simulink model "wlan_phy_rx_pmd"  (Simulink 7.8)
settings: Solver=VariableStepDiscrete  SolverMode=SingleTasking  StartTime=0.0  StopTime=rx_sim.sim_time  RelTol=1e-3
PostLoadFcn:
  | wlan_phy_rx_init
InitFcn:
  | wlan_phy_rx_init
StartFcn:
  | wlan_phy_rx_init
values from neighbouring files (symbols this model uses):
  barker_seq20 = 0.95 * circshift(barker_seq20, [0 4]) ./ max(abs(barker_seq20))    [wlan_phy_rx_init.m]
  bit_scrambler_lfsr_bytes = sum(bit_scrambler_lfsr_bytes .* repmat(2.^[0:7], 127, 1),2)    [wlan_phy_rx_init.m]
  longCorr_coef_i = [3*real(longCorr_coef)]    [wlan_phy_rx_init.m]
  longCorr_coef_q = [3*imag(longCorr_coef)]    [wlan_phy_rx_init.m]
  MAX_CP_LEN = 32    [wlan_phy_rx_init.m]
  MAX_NCBPS = NCBPS_11n_64QAM    (= 312)    [wlan_phy_rx_init.m]
  MAX_NUM_BYTES = 2^16-1    (= 65535)    [wlan_phy_rx_init.m]
  MAX_NUM_SC = 64    [wlan_phy_rx_init.m]
  MAX_NUM_SYMS = 600    [wlan_phy_rx_init.m]
  NDBPS_11a_BPSK12 = 24    [wlan_phy_rx_init.m]
  preFFT_sampBuff_numSamps = 4*MAX_NUM_SC    (= 256)    [wlan_phy_rx_init.m]
  rx_sim = struct()    [wlan_phy_rx_init.m]
  scr_ind_rev = zeros(1,128)    [wlan_phy_rx_init.m]
undefined symbols (not defined in the file or in its folder): gcbh, mcs_rom_11ag, mcs_rom_11n, wlan_rx_deinterleave_rom_gen, xlBlockLoadCallback, xlGetNormalizedPeriod, xlipgetpartsetting
libraries: xbsIndex_r4 x4943, xbsEDKLib_r4 x1
(part 11 of 28)

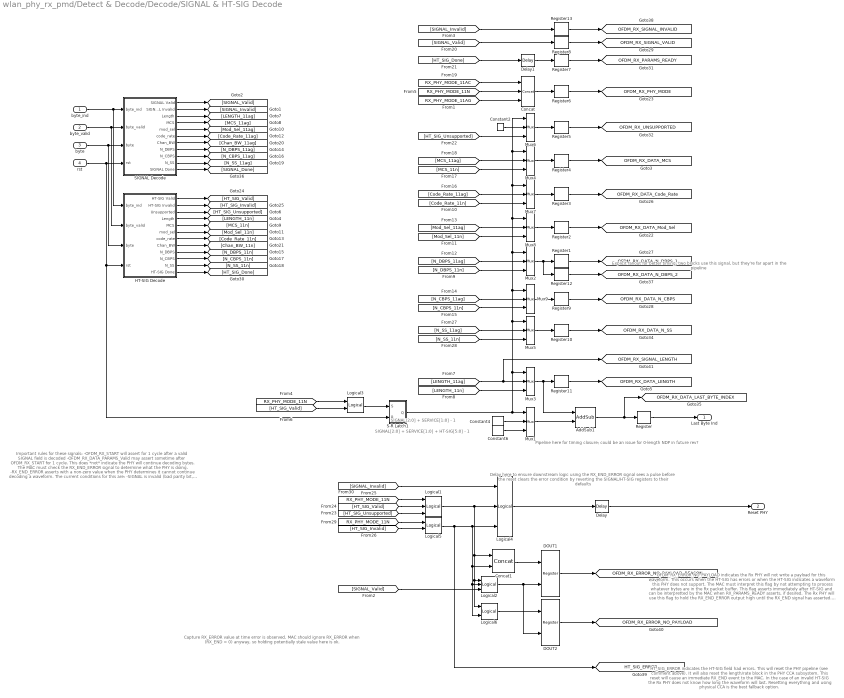
== system wlan_phy_rx_pmd/Detect & Decode/Decode/SIGNAL & HT-SIG Decode ==
  note: SIGNAL[2:0] + SERVICE[1:0] - 1
  note: SIGNAL[2:0] + SERVICE[1:0] +
      | HT-SIG[5:0] - 1
  note: OFDM_RX_ERROR_NO_PAYLOAD indicates the Rx PHY
      | will not write a payload for this waveform. This occurs when
      | the HT-SIG has errors or when the HT-SIG indicates a
      | waveform this PHY does not support. The MAC must interpret
      | this flag by not attempting to process whatever bytes are in
      | the Rx packet buffer.
      |
      | This flag asserts immediately after HT-SIG and can be interpretted
      | by the MAC when RX_PARAMS_READY asserts, if desired.
      |
      | The Rx PHY will use this flag to hold the RX_END_ERROR output
      | high until the RX_END signal has asserted. The MAC uses RX_END
      | to qualify RX_END_ERROR.
  note: Important rules for these signals:
      | -OFDM_RX_START will assert for 1 cycle after a valid SIGNAL field is decoded
      | -OFDM_RX_DATA_PARAMS_Valid may assert sometime after OFDM_RX_START for 1 cycle. This
      |   does *not* indicate the PHY will continue decoding bytes. The MAC must check the RX_END_ERROR
      |   signal to determine what the PHY is doing.
      | -RX_END_ERROR asserts with a non-zero value when the PHY determines it cannot continue decoding
      |   a waveform. The current conditions for this are:
      |    -SIGNAL is invalid (bad parity bit, invalid RATE or LENGTH value)
      |    -HT-SIG is invalid (bad MCS, bad TAIL or bad CRC)
      |    -HT-SIG.MCS is unsupported
  note: Delay here to ensure downstream logic using
      | the RX_END_ERROR signal sees a pulse before
      | the reset clears the error condition by reverting
      | the SIGNAL/HT-SIG registers to their defaults
  note: Capture RX_ERROR value at time error is observed. MAC should
      | ignore RX_ERROR when (RX_END = 0) anyway, so
      | holding potentially stale value here is ok.
  note: Pipeline here for timing closure; could be
      | an issue for 0-length NDP in future rev?
  note: Explicit fanout for better timing; two blocks
      | use this signal, but they're far apart in the pipeline
  note: HT_SIG_ERROR indicates the HT-SIG field had errors. This will reset
      | the PHY pipeline (see comment above). It will also reset the length/rate
      | block in the PHY CCA subsystem. This reset will cause an immediate RX_END
      | event to the MAC. In the case of an invalid HT-SIG the Rx PHY does not know
      | how long the waveform will last. Resetting everything and using physical CCA
      | is the best fallback option.
  byte_ind : Inport
  byte_valid : Inport  Port=2
  byte : Inport  Port=3
  rst : Inport  Port=4
  AddSub1 : xbsIndex_r4/AddSub  mode=Addition  use_carryin=off  use_carryout=off  en=off  latency=0  precision=Full  arith_type="Signed  (2's comp)"  n_bits=ceil(log2(MAX_NUM_BYTES))  bin_pt=0  quantization=Truncate  overflow=Wrap  dbl_ovrd=off  use_behavioral_HDL=off  hw_selection=Fabric  pipelined=off  block_type=addsub
  Concat : xbsIndex_r4/Concat  num_inputs=3  dbl_ovrd=off  block_type=concat
  Concat1 : xbsIndex_r4/Concat  num_inputs=2  dbl_ovrd=off  block_type=concat
  Constant2 : xbsIndex_r4/Constant  const=0  gui_display_data_type=Boolean  arith_type=Boolean  n_bits=2  bin_pt=0  preci_type=Single  exp_width=8  frac_width=24  explicit_period=off  period=1  equ=P=C  opselect=C  inp2=PCIN>>17  opr=+  inp1=P  carry=CIN  dbl_ovrd=off  block_type=constant
  Constant4 : xbsIndex_r4/Constant  const=4  gui_display_data_type=Fixed-point  arith_type=Unsigned  n_bits=3  bin_pt=0  preci_type=Single  exp_width=8  frac_width=24  explicit_period=off  period=1  equ=P=C  opselect=C  inp2=PCIN>>17  opr=+  inp1=P  carry=CIN  dbl_ovrd=off  block_type=constant
  Constant6 : xbsIndex_r4/Constant  const=4+6  gui_display_data_type=Fixed-point  arith_type=Unsigned  n_bits=4  bin_pt=0  preci_type=Single  exp_width=8  frac_width=24  explicit_period=off  period=1  equ=P=C  opselect=C  inp2=PCIN>>17  opr=+  inp1=P  carry=CIN  dbl_ovrd=off  block_type=constant
  DOUT1 : xbsIndex_r4/Register  init=0  rst=off  en=on  dbl_ovrd=off  block_type=register
  DOUT2 : xbsIndex_r4/Register  init=0  rst=off  en=on  dbl_ovrd=off  block_type=register
  Delay : xbsIndex_r4/Delay  rst=off  infoeditControl=Selection of Reset will increase slice count due to use of real FFs and instead of SRLs  en=off  latency=4  dbl_ovrd=off  reg_retiming=off  block_type=delay
  Delay1 : xbsIndex_r4/Delay  rst=off  infoeditControl=Selection of Reset will increase slice count due to use of real FFs and instead of SRLs  en=off  latency=1  dbl_ovrd=off  reg_retiming=off  block_type=delay
  HT-SIG Decode : Subsystem
  Logical1 : xbsIndex_r4/Logical  logical_function=AND  inputs=3  en=off  latency=0  precision=Full  arith_type=Unsigned  n_bits=16  bin_pt=0  align_bp=on  dbl_ovrd=off  block_type=logical
  Logical2 : xbsIndex_r4/Logical  logical_function=OR  inputs=3  en=off  latency=0  precision=Full  arith_type=Unsigned  n_bits=16  bin_pt=0  align_bp=on  dbl_ovrd=off  block_type=logical
  Logical3 : xbsIndex_r4/Logical  logical_function=AND  inputs=2  en=off  latency=0  precision=Full  arith_type=Unsigned  n_bits=16  bin_pt=0  align_bp=on  dbl_ovrd=off  block_type=logical
  Logical4 : xbsIndex_r4/Logical  logical_function=OR  inputs=3  en=off  latency=0  precision=Full  arith_type=Unsigned  n_bits=16  bin_pt=0  align_bp=on  dbl_ovrd=off  block_type=logical
  Logical5 : xbsIndex_r4/Logical  logical_function=AND  inputs=2  en=off  latency=0  precision=Full  arith_type=Unsigned  n_bits=16  bin_pt=0  align_bp=on  dbl_ovrd=off  block_type=logical
  Logical6 : xbsIndex_r4/Logical  logical_function=OR  inputs=2  en=off  latency=0  precision=Full  arith_type=Unsigned  n_bits=16  bin_pt=0  align_bp=on  dbl_ovrd=off  block_type=logical
  Mux{1..9} (9 alike) : xbsIndex_r4/Mux  inputs=2  en=off  latency=0  precision=Full  arith_type=Unsigned  n_bits=16  bin_pt=14  quantization=Truncate  overflow=Wrap  dbl_ovrd=off  block_type=mux
  Register{,1..13} (14 alike) : xbsIndex_r4/Register  init=0  rst=off  en=off  dbl_ovrd=off  block_type=register
  S-R Latch1 : Subsystem
  SIGNAL Decode : Subsystem
  Last Byte Ind : Outport
  Reset PHY : Outport  Port=2
  tag scope: HT_SIG_ERROR=global, OFDM_RX_DATA_Code_Rate=global, OFDM_RX_DATA_LAST_BYTE_INDEX=global, OFDM_RX_DATA_LENGTH=global, OFDM_RX_DATA_MCS=global, OFDM_RX_DATA_Mod_Sel=global, OFDM_RX_DATA_N_CBPS=global, OFDM_RX_DATA_N_DBPS_1=global, OFDM_RX_DATA_N_DBPS_2=global, OFDM_RX_DATA_N_SS=global, OFDM_RX_ERROR_NO_PAYLOAD=global, OFDM_RX_ERROR_NO_PAYLOAD_REASON=global, OFDM_RX_PARAMS_READY=global, OFDM_RX_PHY_MODE=global, OFDM_RX_SIGNAL_INVALID=global, OFDM_RX_SIGNAL_LENGTH=global, OFDM_RX_SIGNAL_VALID=global, OFDM_RX_UNSUPPORTED=global
  wires:
  byte_ind/1 -> SIGNAL Decode/1(byte_ind), HT-SIG Decode/1(byte_ind)
  byte_valid/1 -> SIGNAL Decode/2(byte_valid), HT-SIG Decode/2(byte_valid)
  byte/1 -> SIGNAL Decode/3(byte), HT-SIG Decode/3(byte)
  rst/1 -> SIGNAL Decode/4(rst), HT-SIG Decode/4(rst), S-R Latch1/2(R)
  SIGNAL Decode/3(Length) -> [LENGTH_11ag]
  SIGNAL Decode/4(MCS) -> [MCS_11ag]
  SIGNAL Decode/5(mod_sel) -> [Mod_Sel_11ag]
  SIGNAL Decode/1(SIGNAL Valid) -> [SIGNAL_Valid]
  SIGNAL Decode/2(SIGNAL Invalid) -> [SIGNAL_Invalid]
  SIGNAL Decode/6(code_rate) -> [Code_Rate_11ag]
  SIGNAL Decode/7(Chan_BW) -> [Chan_BW_11ag]
  SIGNAL Decode/8(N_DBPS) -> [N_DBPS_11ag]
  SIGNAL Decode/9(N_CBPS) -> [N_CBPS_11ag]
  SIGNAL Decode/10(N_SS) -> [N_SS_11ag]
  HT-SIG Decode/1(HT-SIG Valid) -> [HT_SIG_Valid]
  HT-SIG Decode/2(HT-SIG Invalid) -> [HT_SIG_Invalid]
  HT-SIG Decode/3(Unsupported) -> [HT_SIG_Unsupported]
  HT-SIG Decode/4(Length) -> [LENGTH_11n]
  HT-SIG Decode/5(MCS) -> [MCS_11n]
  HT-SIG Decode/6(mod_sel) -> [Mod_Sel_11n]
  HT-SIG Decode/7(code_rate) -> [Code_Rate_11n]
  HT-SIG Decode/8(Chan_BW) -> [Chan_BW_11n]
  HT-SIG Decode/9(N_DBPS) -> [N_DBPS_11n]
  HT-SIG Decode/10(N_CBPS) -> [N_CBPS_11n]
  HT-SIG Decode/11(N_SS) -> [N_SS_11n]
  Mux3/1 -> AddSub1/1, Register11/1
  AddSub1/1 -> [OFDM_RX_DATA_LAST_BYTE_INDEX], Register/1
  [LENGTH_11ag] -> Mux3/2, [OFDM_RX_SIGNAL_LENGTH]
  [LENGTH_11n] -> Mux3/3
  [Code_Rate_11ag] -> Mux7/2
  [Code_Rate_11n] -> Mux7/3
  Mux7/1 -> Register3/1
  [Mod_Sel_11ag] -> Mux8/2
  [Mod_Sel_11n] -> Mux8/3
  Mux8/1 -> Register2/1
  [N_DBPS_11ag] -> Mux2/2
  [N_DBPS_11n] -> Mux2/3
  Mux2/1 -> Register1/1, Register12/1
  [N_CBPS_11ag] -> Mux9/2
  [N_CBPS_11n] -> Mux9/3
  Mux9/1 -> Register9/1
  Mux1/1 -> AddSub1/2
  Constant4/1 -> Mux1/2
  Constant6/1 -> Mux1/3
  Logical3/1 -> S-R Latch1/1(S)
  S-R Latch1/1(Q) -> Mux1/1, Mux3/1, Mux9/1, Mux2/1, Mux8/1, Mux7/1, Mux4/1, Mux6/1, Mux5/1
  [MCS_11ag] -> Mux4/2
  [MCS_11n] -> Mux4/3
  Mux4/1 -> Register4/1
  [RX_PHY_MODE_11AC] -> Concat/1
  [RX_PHY_MODE_11N] -> Concat/2
  [RX_PHY_MODE_11AG] -> Concat/3
  Concat/1 -> Register6/1
  [SIGNAL_Valid] -> Register8/1
  HT-SIG Decode/12(HT-SIG Done) -> [HT_SIG_Done]
  [HT_SIG_Done] -> Delay1/1
  Delay1/1 -> Register7/1
  [RX_PHY_MODE_11N] -> Logical1/1
  [HT_SIG_Valid] -> Logical1/2
  [SIGNAL_Invalid] -> Logical4/1
  Logical5/1 -> Logical4/3, Concat1/2, Logical2/2, Logical6/2, [HT_SIG_ERROR]
  Logical4/1 -> Delay/1
  [N_SS_11ag] -> Mux5/2
  [N_SS_11n] -> Mux5/3
  Mux5/1 -> Register10/1
  [HT_SIG_Unsupported] -> Mux6/3
  Constant2/1 -> Mux6/2
  Mux6/1 -> Register5/1
  [RX_PHY_MODE_11N] -> Logical5/1
  [HT_SIG_Invalid] -> Logical5/2
  Logical1/1 -> Logical4/2, Concat1/1, Logical2/1, Logical6/1
  [HT_SIG_Unsupported] -> Logical1/3
  SIGNAL Decode/11(SIGNAL Done) -> [SIGNAL_Done]
  Concat1/1 -> DOUT1/1
  Delay/1 -> Reset PHY/1
  Logical2/1 -> DOUT1/2, DOUT2/2
  DOUT1/1 -> [OFDM_RX_ERROR_NO_PAYLOAD_REASON]
  [SIGNAL_Valid] -> Logical2/3
  Register/1 -> Last Byte Ind/1
  Register1/1 -> [OFDM_RX_DATA_N_DBPS_1]
  Register2/1 -> [OFDM_RX_DATA_Mod_Sel]
  Register3/1 -> [OFDM_RX_DATA_Code_Rate]
  Register4/1 -> [OFDM_RX_DATA_MCS]
  Register5/1 -> [OFDM_RX_UNSUPPORTED]
  Register6/1 -> [OFDM_RX_PHY_MODE]
  Register7/1 -> [OFDM_RX_PARAMS_READY]
  Register8/1 -> [OFDM_RX_SIGNAL_VALID]
  Register9/1 -> [OFDM_RX_DATA_N_CBPS]
  Register10/1 -> [OFDM_RX_DATA_N_SS]
  Register11/1 -> [OFDM_RX_DATA_LENGTH]
  Register12/1 -> [OFDM_RX_DATA_N_DBPS_2]
  [SIGNAL_Invalid] -> Register13/1
  Register13/1 -> [OFDM_RX_SIGNAL_INVALID]
  [RX_PHY_MODE_11N] -> Logical3/1
  [HT_SIG_Valid] -> Logical3/2
  DOUT2/1 -> [OFDM_RX_ERROR_NO_PAYLOAD]
  Logical6/1 -> DOUT2/1

== system wlan_phy_rx_pmd/Detect & Decode/Decode/Safe ToUint8 ==
  note: Simulation-only block: Sets output to 0
      | if input is indeterminate, which occurs at
      | the blackbox outputs before the first reset
  A : Inport
  Convert1 : xbsIndex_r4/Convert  gui_display_data_type=Fixed-point  arith_type=Unsigned  n_bits=8  bin_pt=0  float_type=Single  exp_bits=8  fraction_bits=24  quantization=Truncate  overflow=Wrap  en=off  latency=0  dbl_ovrd=off  pipeline=off  block_type=convert
  Indet Check : Subsystem
  Simulation Multiplexer : xbsIndex_r4/Simulation Multiplexer  sim_sel=1  hw_sel=2  block_type=simmux
      settings: infoedit="Distinguish input subsystems as \"simulation only\" and \"generation only\".  The input specified For Simulation will be used during Simulink simulation.  The input specified For Generation will be used during code generation.  This block will typically be used on the output of a HW Co-Simulation, Black Box, or ModelSim subsystem.<br><br>Hardware Notes: This block costs nothing."
  B : Outport
  wires:
  A/1 -> Indet Check/1(In1), Simulation Multiplexer/2
  Indet Check/1(Out1) -> Simulation Multiplexer/1
  Simulation Multiplexer/1 -> Convert1/1
  Convert1/1 -> B/1

== system wlan_phy_rx_pmd/Detect & Decode/Decode/Stretch ==
  note: Extend duration of reset pulse for use by upstream blocks.
      | A few subsystems require a reset pulse longer than their own
      | latency (like the logic counting input samples to the FFT).
      | Extending the pulse here is more efficient that adding extensions
      | in each subsystem.
  D : Inport
  Constant2 : xbsIndex_r4/Constant  const=160  gui_display_data_type=Fixed-point  arith_type=Unsigned  n_bits=8  bin_pt=0  preci_type=Single  exp_width=8  frac_width=24  explicit_period=off  period=1  equ=P=C  opselect=C  inp2=PCIN>>17  opr=+  inp1=P  carry=CIN  dbl_ovrd=off  block_type=constant
      settings: block_version=VER_STRING_GOES_HERE
  Counter1 : xbsIndex_r4/Counter  cnt_type=Free Running  cnt_to=Inf  operation=Up  start_count=0  cnt_by_val=1  arith_type=Unsigned  n_bits=8  bin_pt=0  load_pin=off  rst=on  en=on  explicit_period=off  period=1  dbl_ovrd=off  use_behavioral_HDL=off  implementation=Fabric  block_type=counter
  Inverter : xbsIndex_r4/Inverter  en=off  latency=0  dbl_ovrd=off  block_type=inv
  Inverter1 : xbsIndex_r4/Inverter  en=off  latency=0  dbl_ovrd=off  block_type=inv
  Logical1 : xbsIndex_r4/Logical  logical_function=OR  inputs=2  en=off  latency=0  precision=Full  arith_type=Unsigned  n_bits=16  bin_pt=0  align_bp=on  dbl_ovrd=off  block_type=logical
  Logical2 : xbsIndex_r4/Logical  logical_function=AND  inputs=2  en=off  latency=0  precision=Full  arith_type=Unsigned  n_bits=16  bin_pt=0  align_bp=on  dbl_ovrd=off  block_type=logical
  Relational3 : xbsIndex_r4/Relational  mode=a>=b  en=off  latency=0  dbl_ovrd=off  block_type=relational
  S-R Latch1 : Subsystem
  Q : Outport
  wires:
  Counter1/1 -> Relational3/1
  Constant2/1 -> Relational3/2
  Inverter/1 -> Counter1/1
  D/1 -> Logical1/1, S-R Latch1/1(S), Inverter1/1
  Relational3/1 -> S-R Latch1/2(R)
  Logical1/1 -> Q/1
  Logical2/1 -> Counter1/2, Inverter/1
  S-R Latch1/1(Q) -> Logical2/1, Logical1/2
  Inverter1/1 -> Logical2/2

== system wlan_phy_rx_pmd/Detect & Decode/Descramble/Descram Byte Calc ==
  note: SERVICE field is used to lookup initial state
      | of Tx scrambler. This state becomes the starting
      | index to the bytewise descrambler logic. This
      | block is a parallel version of the bitwise scrambling
      | specified in the 802.11 spec.
  note: Don't keep the reset signal asserted if a packet
      | happens to end on byte 126 when the next
      | SERVICE field arrives. Without this not/and
      | every 126th packet will be descrambled wrong
      | (when all pkts have the same length)
  data_byte : Inport
  data_valid : Inport  Port=2
  data_ind : Inport  Port=3
  Svc byte ind : Inport  Port=4
  3LSB1 : xbsIndex_r4/Slice  nbits=3  boolean_output=off  mode=Lower Bit Location + Width  bit1=0  base1=MSB of Input  bit0=0  base0=LSB of Input  dbl_ovrd=off  block_type=slice
  4MSB1 : xbsIndex_r4/Slice  nbits=4  boolean_output=off  mode=Upper Bit Location + Width  bit1=0  base1=MSB of Input  bit0=0  base0=LSB of Input  dbl_ovrd=off  block_type=slice
  Concat1 : xbsIndex_r4/Concat  num_inputs=2  dbl_ovrd=off  block_type=concat
  Constant1 : xbsIndex_r4/Constant  const=126  gui_display_data_type=Fixed-point  arith_type=Unsigned  n_bits=7  bin_pt=0  preci_type=Single  exp_width=8  frac_width=24  explicit_period=off  period=1  equ=P=C  opselect=C  inp2=PCIN>>17  opr=+  inp1=P  carry=CIN  dbl_ovrd=off  block_type=constant
  Constant2 : xbsIndex_r4/Constant  const=0  gui_display_data_type=Fixed-point  arith_type=Unsigned  n_bits=8  bin_pt=0  preci_type=Single  exp_width=8  frac_width=24  explicit_period=off  period=1  equ=P=C  opselect=C  inp2=PCIN>>17  opr=+  inp1=P  carry=CIN  dbl_ovrd=off  block_type=constant
  Counter : xbsIndex_r4/Counter  cnt_type=Free Running  cnt_to=126  operation=Up  start_count=0  cnt_by_val=1  arith_type=Unsigned  n_bits=7  bin_pt=0  load_pin=on  rst=on  en=on  explicit_period=off  period=1  dbl_ovrd=off  use_behavioral_HDL=off  implementation=Fabric  block_type=counter
  Delay{1..4} (4 alike) : xbsIndex_r4/Delay  rst=off  infoeditControl=Selection of Reset will increase slice count due to use of real FFs and instead of SRLs  en=off  latency=1  dbl_ovrd=off  reg_retiming=off  block_type=delay
  Delay5 : xbsIndex_r4/Delay  rst=off  infoeditControl=Selection of Reset will increase slice count due to use of real FFs and instead of SRLs  en=off  latency=3  dbl_ovrd=off  reg_retiming=off  block_type=delay
  Descrm Ind : Scope  NumInputPorts=8
      settings: TimeRange=8000  YMin=70~102~50~-1~-5~-5~-5~-5  YMax=210~105~135~1~5~5~5~5  SaveName=ScopeData19
  Gateway Out{1..8} (8 alike) : xbsIndex_r4/Gateway Out  inherit_from_input=off  hdl_port=off  timing_constraint=None  locs_specified=off  LOCs={}  UseAsDAC=off  DACChannel='1'  block_type=gatewayout
  Inverter : xbsIndex_r4/Inverter  en=off  latency=0  dbl_ovrd=off  block_type=inv
  LFSR Init : Subsystem
  LFSR Init State LUT : xbsIndex_r4/ROM  depth=length(scr_ind_rev)  initVector=scr_ind_rev  distributed_mem=Distributed memory  rst=off  init_reg=0  en=off  latency=0  gui_display_data_type=Fixed-point  arith_type=Unsigned  n_bits=7  bin_pt=0  preci_type=Single  exp_width=8  frac_width=24  dbl_ovrd=off  optimize=Area  use_rpm=on  block_type=sprom
  LFSR by Byte : xbsIndex_r4/ROM  depth=length(bit_scrambler_lfsr_bytes)  initVector=bit_scrambler_lfsr_bytes  distributed_mem=Block RAM  rst=off  init_reg=0  en=off  latency=1  gui_display_data_type=Fixed-point  arith_type=Unsigned  n_bits=8  bin_pt=0  preci_type=Single  exp_width=8  frac_width=24  dbl_ovrd=off  optimize=Area  use_rpm=on  block_type=sprom
  Logical : xbsIndex_r4/Logical  logical_function=AND  inputs=2  en=off  latency=0  precision=Full  arith_type=Unsigned  n_bits=16  bin_pt=0  align_bp=on  dbl_ovrd=off  block_type=logical
  Logical1 : xbsIndex_r4/Logical  logical_function=OR  inputs=2  en=off  latency=0  precision=Full  arith_type=Unsigned  n_bits=16  bin_pt=0  align_bp=on  dbl_ovrd=off  block_type=logical
  Logical2 : xbsIndex_r4/Logical  logical_function=AND  inputs=3  en=off  latency=0  precision=Full  arith_type=Unsigned  n_bits=16  bin_pt=0  align_bp=on  dbl_ovrd=off  block_type=logical
  Mux : xbsIndex_r4/Mux  inputs=2  en=off  latency=0  precision=Full  arith_type=Unsigned  n_bits=16  bin_pt=14  quantization=Truncate  overflow=Wrap  dbl_ovrd=off  block_type=mux
  Relational : xbsIndex_r4/Relational  mode=a=b  en=off  latency=0  dbl_ovrd=off  block_type=relational
  Relational1 : xbsIndex_r4/Relational  mode=a=b  en=off  latency=0  dbl_ovrd=off  block_type=relational
  Relational2 : xbsIndex_r4/Relational  mode=a>=b  en=off  latency=0  dbl_ovrd=off  block_type=relational
  SERVICE : xbsIndex_r4/Register  init=0  rst=off  en=on  dbl_ovrd=off  block_type=register
  Descram Val : Outport
  wires:
  Logical2/1 -> Counter/3
  Relational1/1 -> Logical2/1
  Delay4/1 -> Gateway Out3/1
  Delay2/1 -> Delay4/1
  Concat1/1 -> Gateway Out1/1, Counter/2
  Gateway Out4/1 -> Descrm Ind/6
  4MSB1/1 -> Concat1/2
  3LSB1/1 -> Concat1/1
  Gateway Out3/1 -> Descrm Ind/5
  Gateway Out2/1 -> Descrm Ind/4
  Gateway Out1/1 -> Descrm Ind/3
  Gateway Out6/1 -> Descrm Ind/2
  Gateway Out5/1 -> Descrm Ind/1
  Counter/1 -> LFSR by Byte/1, Gateway Out2/1, Relational1/1
  Constant1/1 -> Relational1/2
  Delay1/1 -> Logical1/2, Logical2/3, Delay2/1
  Logical1/1 -> Counter/4
  Delay3/1 -> Logical1/1, Counter/1, Inverter/1
  LFSR Init State LUT/1 -> 4MSB1/1, 3LSB1/1, Gateway Out6/1
  LFSR by Byte/1 -> Gateway Out4/1, Mux/3
  data_ind/1 -> Relational/1, Relational2/1, Gateway Out8/1
  data_valid/1 -> Delay1/1, Logical/1
  data_byte/1 -> SERVICE/1
  LFSR Init/1(I) -> Gateway Out5/1, LFSR Init State LUT/1
  SERVICE/1 -> LFSR Init/1(B)
  Logical/1 -> Delay3/1, SERVICE/2
  Relational/1 -> Logical/2
  Svc byte ind/1 -> Relational/2, Relational2/2
  Mux/1 -> Descram Val/1
  Relational2/1 -> Delay5/1
  Constant2/1 -> Mux/2
  Gateway Out7/1 -> Descrm Ind/7
  Gateway Out8/1 -> Descrm Ind/8
  Delay5/1 -> Mux/1, Gateway Out7/1
  Inverter/1 -> Logical2/2

== system wlan_phy_rx_pmd/Detect & Decode/Soft Demod/16QAM Soft De-Map ==
  note: 16-QAM Demap:
      | Each sym is [b0 b1 b2 b3]
      | b0 = 1 if (I > 0)
      | b1 = 1 if(abs(I) < 2/sqrt(10))
      | b2 = 1 if(Q > 0)
      | b3 = 1 if(abs(Q) < 2/sqrt(10))
  I : Inport
  Q : Inport  Port=2
  16QAM Demap : Scope  NumInputPorts=8
      settings: TimeRange=20000  YMin=0~-1~0~0~-1~0~-1~-1  YMax=1~1~1~1~1~1~1~1  SaveName=ScopeData26
  Register1 : xbsIndex_r4/Register  init=0  rst=off  en=off  dbl_ovrd=off  block_type=register
  Register16 : xbsIndex_r4/Register  init=0  rst=off  en=off  dbl_ovrd=off  block_type=register
  b1/b3 map1 : Subsystem
  b1/b3 map2 : Subsystem
  b0 : Outport
  b1 : Outport  Port=2
  b2 : Outport  Port=3
  b3 : Outport  Port=4
  wires:
  I/1 -> b1/b3 map1/1(IQ), Register16/1
  ? -> 16QAM Demap/6  "Ready"
  ? -> 16QAM Demap/5  "Vout"
  ? -> 16QAM Demap/4  "Mux Sel"
  ? -> 16QAM Demap/3  "Q In"
  ? -> 16QAM Demap/2  "I In"
  ? -> 16QAM Demap/1  "valid in"
  Q/1 -> b1/b3 map2/1(IQ), Register1/1
  b1/b3 map1/1(b) -> b1/1
  b1/b3 map2/1(b) -> b3/1
  Register16/1 -> b0/1
  Register1/1 -> b2/1

== system wlan_phy_rx_pmd/Detect & Decode/Soft Demod/64QAM Soft De-Map ==
  note: 64-QAM Demap:
      | Each sym is [b0 b1 b2 b3 b4 b5]
      | b0 = 1 if (I > 0)
      | b1 = 1 if(abs(I) < 4/sqrt(42))
      | b2 = 1 if(2/sqrt(42) < abs(I) < 6/sqrt(42))
      |
      | b3 = 1 if(Q > 0)
      | b4 = 1 if(abs(Q) < 4/sqrt(42))
      | b5 = 1 if(2/sqrt(42) < abs(Q) < 6/sqrt(42))
  I : Inport
  Q : Inport  Port=2
  64QAM Demap : Scope  NumInputPorts=8
      settings: TimeRange=20000  YMin=0~-1~0~0~-1~0~-1~-1  YMax=1~1~1~1~1~1~1~1  SaveName=ScopeData26
  Bus Creator : BusCreator  Inputs=3
  Bus Creator1 : BusCreator  Inputs=3
  Gateway Out{1..3,5..9} (8 alike) : xbsIndex_r4/Gateway Out  inherit_from_input=off  hdl_port=off  timing_constraint=None  locs_specified=off  LOCs={}  UseAsDAC=off  DACChannel='1'  block_type=gatewayout
  Register1 : xbsIndex_r4/Register  init=0  rst=off  en=off  dbl_ovrd=off  block_type=register
  Register16 : xbsIndex_r4/Register  init=0  rst=off  en=off  dbl_ovrd=off  block_type=register
  b1/b4 map : Subsystem
  b1/b4 map1 : Subsystem
  b2/b5 map : Subsystem
  b2/b5 map1 : Subsystem
  b0 : Outport
  b1 : Outport  Port=2
  b2 : Outport  Port=3
  b3 : Outport  Port=4
  b4 : Outport  Port=5
  b5 : Outport  Port=6
  wires:
  b1/b4 map/1(b) -> b1/1, Gateway Out5/1
  b2/b5 map/1(b) -> b2/1, Gateway Out6/1
  I/1 -> b1/b4 map/1(IQ), b2/b5 map/1(IQ), Gateway Out3/1, Register16/1
  Q/1 -> Gateway Out2/1, b2/b5 map1/1(IQ), b1/b4 map1/1(IQ), Register1/1
  b1/b4 map1/1(b) -> b4/1, Gateway Out8/1
  b2/b5 map1/1(b) -> b5/1, Gateway Out9/1
  Bus Creator/1 -> 64QAM Demap/2  "I Bits"
  Gateway Out3/1 -> 64QAM Demap/1  "I In"
  Gateway Out2/1 -> 64QAM Demap/3  "Q In"
  Bus Creator1/1 -> 64QAM Demap/4  "Q Bits"
  Gateway Out1/1 -> Bus Creator/1  "I Bits"
  Gateway Out5/1 -> Bus Creator/2
  Gateway Out6/1 -> Bus Creator/3
  Gateway Out7/1 -> Bus Creator1/1
  Gateway Out8/1 -> Bus Creator1/2
  Gateway Out9/1 -> Bus Creator1/3
  Register16/1 -> b0/1, Gateway Out1/1
  Register1/1 -> b3/1, Gateway Out7/1

== system wlan_phy_rx_pmd/Detect & Decode/Soft Demod/BPSK Soft De-Map ==
  note: BPSK Demap:
      | Each sym is [b0]
      | b0 = 1 if (I > 0)
  note: QBPSK Demap:
      | Each sym is [b0]
      | b0 = 1 if (Q > 0)
  rot90 : Inport
  I : Inport  Port=2
  Q : Inport  Port=3
  Add : Sum  Inputs=+-
  BPSK Demap : Scope  NumInputPorts=8
      settings: TimeRange=20000  YMin=0~-1~0~0~-1~0~-1~-1  YMax=1~1~1~1~1~1~1~1  SaveName=ScopeData26
  Gateway Out{1..4} (4 alike) : xbsIndex_r4/Gateway Out  inherit_from_input=off  hdl_port=off  timing_constraint=None  locs_specified=off  LOCs={}  UseAsDAC=off  DACChannel='1'  block_type=gatewayout
  Mux4 : xbsIndex_r4/Mux  inputs=2  en=off  latency=1  precision=Full  arith_type=Unsigned  n_bits=16  bin_pt=14  quantization=Truncate  overflow=Wrap  dbl_ovrd=off  block_type=mux
  Sign : Signum
  Sign1 : Signum
  b0 : Outport
  wires:
  rot90/1 -> Mux4/1, Gateway Out2/1
  I/1 -> Mux4/2, Gateway Out3/1
  Q/1 -> Mux4/3, Gateway Out1/1
  Mux4/1 -> b0/1, Gateway Out4/1
  Gateway Out3/1 -> BPSK Demap/1  "I In"
  Gateway Out1/1 -> BPSK Demap/2  "Q In"
  Gateway Out2/1 -> BPSK Demap/3  "Mux sel"
  Gateway Out4/1 -> BPSK Demap/4  "Out 9_7"
  ? -> BPSK Demap/5  "Out 8_7"
  ? -> BPSK Demap/6, Sign/1
  ? -> BPSK Demap/7, Sign1/1
  Add/1 -> BPSK Demap/8
  Sign/1 -> Add/1
  Sign1/1 -> Add/2

== system wlan_phy_rx_pmd/Detect & Decode/Soft Demod/QPSK Soft De-Map ==
  note: QPSK Demap:
      | Each sym is [b0 b1]
      | b0 = 1 if (I > 0)
      | b1 = 1 if(Q > 0)
  I : Inport
  Q : Inport  Port=2
  QPSK Demap : Scope  NumInputPorts=8
      settings: TimeRange=20000  YMin=0~-1~0~0~-1~0~-1~-1  YMax=1~1~1~1~1~1~1~1  SaveName=ScopeData26
  Register1 : xbsIndex_r4/Register  init=0  rst=off  en=off  dbl_ovrd=off  block_type=register
  Register16 : xbsIndex_r4/Register  init=0  rst=off  en=off  dbl_ovrd=off  block_type=register
  b0 : Outport
  b1 : Outport  Port=2
  wires:
  Q/1 -> Register1/1
  ? -> QPSK Demap/1  "valid in"
  ? -> QPSK Demap/2  "I In"
  ? -> QPSK Demap/3  "Q In"
  ? -> QPSK Demap/4  "Mux Sel"
  ? -> QPSK Demap/5  "Vout"
  ? -> QPSK Demap/6  "Ready"
  I/1 -> Register16/1
  Register16/1 -> b0/1
  Register1/1 -> b1/1

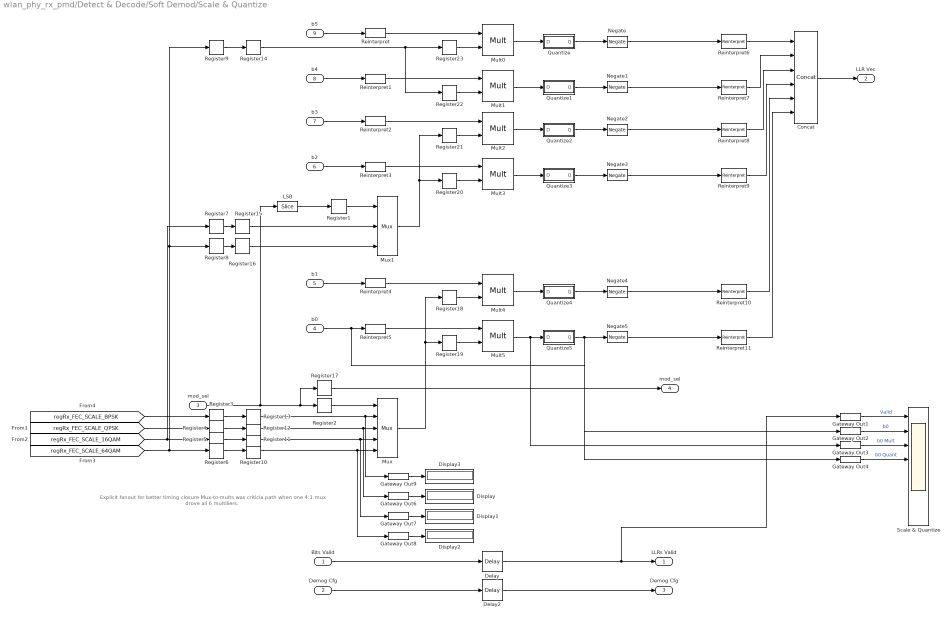
== system wlan_phy_rx_pmd/Detect & Decode/Soft Demod/Scale & Quantize ==
  note: Explicit fanout for better timing closure
      | Mux-to-mults was criticla path when one 4:1 mux
      | drove all 6 multiliers.
  Bits Valid : Inport
  Demog Cfg : Inport  Port=2
  mod_sel : Inport  Port=3
  b0 : Inport  Port=4
  b1 : Inport  Port=5
  b2 : Inport  Port=6
  b3 : Inport  Port=7
  b4 : Inport  Port=8
  b5 : Inport  Port=9
  Concat : xbsIndex_r4/Concat  num_inputs=6  dbl_ovrd=off  block_type=concat
  Delay : xbsIndex_r4/Delay  rst=off  infoeditControl=Selection of Reset will increase slice count due to use of real FFs and instead of SRLs  en=off  latency=1  dbl_ovrd=off  reg_retiming=off  block_type=delay
  Delay2 : xbsIndex_r4/Delay  rst=off  infoeditControl=Selection of Reset will increase slice count due to use of real FFs and instead of SRLs  en=off  latency=1  dbl_ovrd=off  reg_retiming=off  block_type=delay
  Display{,1..3} (4 alike) : Display
  Gateway Out{1..4,6..9} (8 alike) : xbsIndex_r4/Gateway Out  inherit_from_input=off  hdl_port=off  timing_constraint=None  locs_specified=off  LOCs={}  UseAsDAC=off  DACChannel='1'  block_type=gatewayout
  LSB : xbsIndex_r4/Slice  nbits=1  boolean_output=off  mode=Lower Bit Location + Width  bit1=0  base1=MSB of Input  bit0=0  base0=LSB of Input  dbl_ovrd=off  block_type=slice
  Mult{0..5} (6 alike) : xbsIndex_r4/Mult  precision=User Defined  arith_type="Signed  (2's comp)"  n_bits=13  bin_pt=0  quantization=Truncate  overflow=Saturate  en=off  latency=1  dbl_ovrd=off  use_behavioral_HDL=off  opt=Speed  use_embedded=on  optimum_pipeline=off  pipeline=on  use_rpm=on  placement_style=Triangular  block_type=mult
  Mux : xbsIndex_r4/Mux  inputs=4  en=off  latency=1  precision=Full  arith_type=Unsigned  n_bits=16  bin_pt=14  quantization=Truncate  overflow=Wrap  dbl_ovrd=off  block_type=mux
  Mux1 : xbsIndex_r4/Mux  inputs=2  en=off  latency=1  precision=Full  arith_type=Unsigned  n_bits=16  bin_pt=14  quantization=Truncate  overflow=Wrap  dbl_ovrd=off  block_type=mux
  Negate{,1..5} (6 alike) : xbsIndex_r4/Negate  precision=User Defined  arith_type="Signed  (2's comp)"  n_bits=4  bin_pt=0  quantization=Truncate  overflow=Saturate  en=off  latency=0  dbl_ovrd=off  block_type=negate
  Quantize : Subsystem
  Quantize1 : Subsystem
  Quantize2 : Subsystem
  Quantize3 : Subsystem
  Quantize4 : Subsystem
  Quantize5 : Subsystem
  Register{1..23} (23 alike) : xbsIndex_r4/Register  init=0  rst=off  en=off  dbl_ovrd=off  block_type=register
  Reinterpret{,1..5} (6 alike) : xbsIndex_r4/Reinterpret  force_arith_type=off  arith_type="Signed  (2's comp)"  force_bin_pt=on  bin_pt=0  block_type=reinterpret
  Reinterpret{6..11} (6 alike) : xbsIndex_r4/Reinterpret  force_arith_type=on  arith_type=Unsigned  force_bin_pt=on  bin_pt=0  block_type=reinterpret
  Scale & Quantize : Scope  NumInputPorts=8
      settings: TimeRange=9000  YMin=0~0~0~0~-1~0~41.76470588235293~-0.1  YMax=2~1~1~1000~1~1~60.58823529411768~1.1  SaveName=ScopeData51
  LLRs Valid : Outport
  LLR Vec : Outport  Port=2
  " Demog Cfg" : Outport  Port=3
  " mod_sel" : Outport  Port=4
  wires:
  [regRx_FEC_SCALE_QPSK] -> Register4/1
  [regRx_FEC_SCALE_16QAM] -> Register5/1, Register7/1
  [regRx_FEC_SCALE_64QAM] -> Register6/1, Register8/1, Register9/1
  b5/1 -> Reinterpret/1
  Mult0/1 -> Quantize/1(D)
  Quantize/1(Q) -> Negate/1
  Bits Valid/1 -> Delay/1
  Delay/1 -> LLRs Valid/1, Gateway Out1/1
  Reinterpret/1 -> Mult0/1
  Negate/1 -> Reinterpret6/1
  Gateway Out8/1 -> Display2/1
  Gateway Out7/1 -> Display1/1
  Gateway Out6/1 -> Display/1
  b4/1 -> Reinterpret1/1
  Mult1/1 -> Quantize1/1(D)
  Quantize1/1(Q) -> Negate1/1
  Reinterpret1/1 -> Mult1/1
  Negate1/1 -> Reinterpret7/1
  b3/1 -> Reinterpret2/1
  Mult2/1 -> Quantize2/1(D)
  Quantize2/1(Q) -> Negate2/1
  Reinterpret2/1 -> Mult2/1
  Negate2/1 -> Reinterpret8/1
  b2/1 -> Reinterpret3/1
  Mult3/1 -> Quantize3/1(D)
  Quantize3/1(Q) -> Negate3/1
  Reinterpret3/1 -> Mult3/1
  Negate3/1 -> Reinterpret9/1
  b1/1 -> Reinterpret4/1
  Mult4/1 -> Quantize4/1(D)
  Quantize4/1(Q) -> Negate4/1
  Reinterpret4/1 -> Mult4/1
  Negate4/1 -> Reinterpret10/1
  b0/1 -> Reinterpret5/1, Gateway Out2/1
  Mult5/1 -> Quantize5/1(D), Gateway Out3/1
  Quantize5/1(Q) -> Negate5/1, Gateway Out4/1
  Reinterpret5/1 -> Mult5/1
  Negate5/1 -> Reinterpret11/1
  Demog Cfg/1 -> Delay2/1
  Delay2/1 -> " Demog Cfg"/1
  Concat/1 -> LLR Vec/1
  Reinterpret6/1 -> Concat/1
  Reinterpret7/1 -> Concat/2
  Reinterpret8/1 -> Concat/3
  Reinterpret9/1 -> Concat/4
  Reinterpret10/1 -> Concat/5
  Reinterpret11/1 -> Concat/6
  [regRx_FEC_SCALE_BPSK] -> Register3/1
  Gateway Out9/1 -> Display3/1
  Mux/1 -> Register18/1, Register19/1
  mod_sel/1 -> LSB/1, Register2/1, Register17/1
  Register2/1 -> Mux/1
  Mux1/1 -> Register20/1, Register21/1
  Register1/1 -> Mux1/1
  LSB/1 -> Register1/1
  Register3/1 -> Register13/1
  Register4/1 -> Register12/1
  Register5/1 -> Register11/1
  Register6/1 -> Register10/1
  Register7/1 -> Register15/1
  Register8/1 -> Register16/1
  Register9/1 -> Register14/1
  Gateway Out1/1 -> Scale & Quantize/1  "Valid"
  Gateway Out2/1 -> Scale & Quantize/2  "b0"
  Gateway Out3/1 -> Scale & Quantize/3  "b0 Mult"
  Gateway Out4/1 -> Scale & Quantize/4  "b0 Quant"
  Register10/1 -> Gateway Out8/1, Mux/5
  Register11/1 -> Gateway Out7/1, Mux/4
  Register12/1 -> Gateway Out6/1, Mux/3
  Register13/1 -> Gateway Out9/1, Mux/2
  Register14/1 -> Register22/1, Register23/1
  Register15/1 -> Mux1/2
  Register16/1 -> Mux1/3
  Register17/1 -> " mod_sel"/1
  Register18/1 -> Mult4/2
  Register19/1 -> Mult5/2
  Register20/1 -> Mult3/2
  Register21/1 -> Mult2/2
  Register22/1 -> Mult1/2
  Register23/1 -> Mult0/2

== system wlan_phy_rx_pmd/Detect & Decode/Subcarrier Map & Demod Control/Demod Config ==
  note: Capture current PHY mode decision
      | at start of each OFDM sym (subcarrier 0)
      | Every PHY mode sets subcarrier 0 to 0,
      | so one cycle ambiguity about PHY mode
      | at subcarrier 0 is no problem
  note: OFDM Symbols in 11a/g:
      | 0: LTF (BPSK)
      | 1: LTF (BPSK)
      | 2: SIG (BPSK) 48sc
      | 3-N: Data  48sc
  note: OFDM Symbols in 11n SISO:
      | 0: L-LTF (BPSK)
      | 1: L-LTF (BPSK)
      | 2: L-SIG (BPSK) 48sc
      | 3: HT-SIG1 (QBPSK) 48sc
      | 4: HT-SIG2 (QBPSK) 48sc
      | 5: HT-STF
      | 6: HT-LFT1
      | 7-N: Data 52sc
  note: OFDM Symbols in 11ac SISO:
      | 0: L-LTF (BPSK)
      | 1: L-LTF (BPSK)
      | 2: L-SIG (BPSK) 48sc
      | 3: VHT-SIGA1 (BPSK) 48sc
      | 4: VHT-SIGA2 (QBPSK) 48sc
      | 5: VHT-STF
      | 6: VHT-LFT1
      | 7: VHT-SIGB 52sc
      | 8-N: Data 52sc
  sc_ind : Inport
  sym_ind : Inport  Port=2
  Constant1 : xbsIndex_r4/Constant  const=0  gui_display_data_type=Fixed-point  arith_type=Unsigned  n_bits=1  bin_pt=0  preci_type=Single  exp_width=8  frac_width=24  explicit_period=off  period=1  equ=P=C  opselect=C  inp2=PCIN>>17  opr=+  inp1=P  carry=CIN  dbl_ovrd=off  block_type=constant
  Constant10 : xbsIndex_r4/Constant  const=3  gui_display_data_type=Fixed-point  arith_type=Unsigned  n_bits=3  bin_pt=0  preci_type=Single  exp_width=8  frac_width=24  explicit_period=off  period=1  equ=P=C  opselect=C  inp2=PCIN>>17  opr=+  inp1=P  carry=CIN  dbl_ovrd=off  block_type=constant
  Constant3 : xbsIndex_r4/Constant  const=4  gui_display_data_type=Fixed-point  arith_type=Unsigned  n_bits=3  bin_pt=0  preci_type=Single  exp_width=8  frac_width=24  explicit_period=off  period=1  equ=P=C  opselect=C  inp2=PCIN>>17  opr=+  inp1=P  carry=CIN  dbl_ovrd=off  block_type=constant
  Constant8 : xbsIndex_r4/Constant  const=7  gui_display_data_type=Fixed-point  arith_type=Unsigned  n_bits=3  bin_pt=0  preci_type=Single  exp_width=8  frac_width=24  explicit_period=off  period=1  equ=P=C  opselect=C  inp2=PCIN>>17  opr=+  inp1=P  carry=CIN  dbl_ovrd=off  block_type=constant
  Constant9 : xbsIndex_r4/Constant  const=2  gui_display_data_type=Fixed-point  arith_type=Unsigned  n_bits=2  bin_pt=0  preci_type=Single  exp_width=8  frac_width=24  explicit_period=off  period=1  equ=P=C  opselect=C  inp2=PCIN>>17  opr=+  inp1=P  carry=CIN  dbl_ovrd=off  block_type=constant
  Demod Config : Scope  NumInputPorts=8
      settings: TimeRange=953.8248648097133  YMin=0~0~0~0~-0.1~-0.1~-0.1~-0.1  YMax=100~30~1~1~1.1~1.1~1.1~1.1  SaveName=ScopeData47
  Gateway Out{1..8} (8 alike) : xbsIndex_r4/Gateway Out  inherit_from_input=off  hdl_port=off  timing_constraint=None  locs_specified=off  LOCs={}  UseAsDAC=off  DACChannel='1'  block_type=gatewayout
  Inverter : xbsIndex_r4/Inverter  en=off  latency=0  dbl_ovrd=off  block_type=inv
  Logical{1..2,4,6} (4 alike) : xbsIndex_r4/Logical  logical_function=OR  inputs=2  en=off  latency=0  precision=Full  arith_type=Unsigned  n_bits=16  bin_pt=0  align_bp=on  dbl_ovrd=off  block_type=logical
  Logical3 : xbsIndex_r4/Logical  logical_function=AND  inputs=2  en=off  latency=0  precision=Full  arith_type=Unsigned  n_bits=16  bin_pt=0  align_bp=on  dbl_ovrd=off  block_type=logical
  Logical5 : xbsIndex_r4/Logical  logical_function=AND  inputs=2  en=off  latency=0  precision=Full  arith_type=Unsigned  n_bits=16  bin_pt=0  align_bp=on  dbl_ovrd=off  block_type=logical
  Logical7 : xbsIndex_r4/Logical  logical_function=AND  inputs=2  en=off  latency=0  precision=Full  arith_type=Unsigned  n_bits=16  bin_pt=0  align_bp=on  dbl_ovrd=off  block_type=logical
  Register : xbsIndex_r4/Register  init=0  rst=off  en=on  dbl_ovrd=off  block_type=register
  Register1 : xbsIndex_r4/Register  init=0  rst=off  en=on  dbl_ovrd=off  block_type=register
  Relational{2..3,9..10} (4 alike) : xbsIndex_r4/Relational  mode=a=b  en=off  latency=0  dbl_ovrd=off  block_type=relational
  Relational7 : xbsIndex_r4/Relational  mode=a<b  en=off  latency=0  dbl_ovrd=off  block_type=relational
  48sc Sym : Outport
  QBPSK Sym : Outport  Port=2
  SIGNAL Sym : Outport  Port=3
  wires:
  Constant10/1 -> Relational10/2
  Constant9/1 -> Relational9/2
  Constant8/1 -> Relational7/2
  sym_ind/1 -> Relational9/1, Relational10/1, Relational3/1, Relational7/1, Gateway Out3/1
  sc_ind/1 -> Relational2/2, Gateway Out1/1
  [RX_PHY_MODE_11N_AC] -> Register/1
  Constant1/1 -> Relational2/1
  Relational2/1 -> Register/2, Register1/2
  [RX_PHY_MODE_11N] -> Register1/1
  Inverter/1 -> Logical1/1
  Register/1 -> [PHY_11N_AC], Gateway Out6/1
  Register1/1 -> [PHY_11N], Gateway Out5/1
  [PHY_11N_AC] -> Inverter/1, Logical3/1, Logical5/1
  Relational7/1 -> Logical1/2
  Logical1/1 -> 48sc Sym/1  "48_Subcarrier_Sym"
  Constant3/1 -> Relational3/2
  Relational3/1 -> Logical2/2, Logical5/2
  Logical2/1 -> Logical3/2
  Relational10/1 -> Logical2/1, Logical7/2
  Logical3/1 -> Logical4/2
  Relational9/1 -> Logical4/1
  Logical4/1 -> SIGNAL Sym/1, Gateway Out7/1  "SIGNAL_Sym"
  [PHY_11N] -> Logical7/1
  Logical7/1 -> Logical6/1
  Logical5/1 -> Logical6/2
  Logical6/1 -> QBPSK Sym/1, Gateway Out8/1  "QBPSK_Sym"
  Gateway Out1/1 -> Demod Config/1  "Sc Ind"
  Gateway Out3/1 -> Demod Config/2  "Sym Ind"
  Gateway Out2/1 -> Demod Config/3  "PHY Mode 11N"
  Gateway Out4/1 -> Demod Config/4  "PHY Mode 11N_AC"
  Gateway Out5/1 -> Demod Config/5  "PHY Mode 11N capt"
  Gateway Out6/1 -> Demod Config/6  "PHY Mode 11N_AC capt"
  [RX_PHY_MODE_11N] -> Gateway Out2/1
  [RX_PHY_MODE_11N_AC] -> Gateway Out4/1
  Gateway Out7/1 -> Demod Config/7  "SIGNAL Sym"
  Gateway Out8/1 -> Demod Config/8  "QBPSK Sym"

== system wlan_phy_rx_pmd/Detect & Decode/Subcarrier Map & Demod Control/Posedge ==
  note: Rising Edge Detection
  D : Inport
  Delay : xbsIndex_r4/Delay  rst=off  infoeditControl=Selection of Reset will increase slice count due to use of real FFs and instead of SRLs  en=off  latency=1  dbl_ovrd=off  reg_retiming=off  block_type=delay
      settings: infoedit=Hardware notes: A delay line is a chain, each link of which is an SRL16 followed by a flip-flop. If register retiming is enabled, the delay line is a chain of flip-flops.  xl_area=[1 1 0 0 0 0 0]  block_version=VER_STRING_GOES_HERE
  Inverter : xbsIndex_r4/Inverter  en=off  latency=0  dbl_ovrd=off  block_type=inv
      settings: xl_area=[0 0 0 0 0 0 0]  block_version=VER_STRING_GOES_HERE
  Logical1 : xbsIndex_r4/Logical  logical_function=AND  inputs=2  en=off  latency=0  precision=Full  arith_type=Unsigned  n_bits=8  bin_pt=2  align_bp=on  dbl_ovrd=off  block_type=logical
      settings: xl_area=[1 0 0 1 0 0 0]  block_version=VER_STRING_GOES_HERE
  Q : Outport
  wires:
  D/1 -> Logical1/2, Delay/1
  Logical1/1 -> Q/1
  Inverter/1 -> Logical1/1
  Delay/1 -> Inverter/1

== system wlan_phy_rx_pmd/Detect & Decode/Subcarrier Map & Demod Control/Posedge1 ==
  note: Rising Edge Detection
  D : Inport
  Delay : xbsIndex_r4/Delay  rst=off  infoeditControl=Selection of Reset will increase slice count due to use of real FFs and instead of SRLs  en=off  latency=1  dbl_ovrd=off  reg_retiming=off  block_type=delay
      settings: infoedit=Hardware notes: A delay line is a chain, each link of which is an SRL16 followed by a flip-flop. If register retiming is enabled, the delay line is a chain of flip-flops.  xl_area=[1 1 0 0 0 0 0]  block_version=VER_STRING_GOES_HERE
  Inverter : xbsIndex_r4/Inverter  en=off  latency=0  dbl_ovrd=off  block_type=inv
      settings: xl_area=[0 0 0 0 0 0 0]  block_version=VER_STRING_GOES_HERE
  Logical1 : xbsIndex_r4/Logical  logical_function=AND  inputs=2  en=off  latency=0  precision=Full  arith_type=Unsigned  n_bits=8  bin_pt=2  align_bp=on  dbl_ovrd=off  block_type=logical
      settings: xl_area=[1 0 0 1 0 0 0]  block_version=VER_STRING_GOES_HERE
  Q : Outport
  wires:
  Delay/1 -> Inverter/1
  Inverter/1 -> Logical1/1
  Logical1/1 -> Q/1
  D/1 -> Delay/1, Logical1/2

== system wlan_phy_rx_pmd/FCS & Pkt Buf/FCS Calc/CRC32 Calc ==
  byte_valid : Inport
  byte : Inport  Port=2
  rst : Inport  Port=3
  24LSB : xbsIndex_r4/Slice  nbits=24  boolean_output=off  mode=Lower Bit Location + Width  bit1=0  base1=MSB of Input  bit0=0  base0=LSB of Input  dbl_ovrd=off  block_type=slice
      settings: infoedit="Extracts a given range of bits from each input sample and presents it at the output.  The output type is ordinarily unsigned with binary point at zero, but can be Boolean when the slice is one bit wide.<P><P>Hardware notes: In hardware this block costs nothing."  block_version=VER_STRING_GOES_HERE
  8MSB : xbsIndex_r4/Slice  nbits=8  boolean_output=off  mode=Upper Bit Location + Width  bit1=0  base1=MSB of Input  bit0=0  base0=LSB of Input  dbl_ovrd=off  block_type=slice
      settings: infoedit="Extracts a given range of bits from each input sample and presents it at the output.  The output type is ordinarily unsigned with binary point at zero, but can be Boolean when the slice is one bit wide.<P><P>Hardware notes: In hardware this block costs nothing."  block_version=VER_STRING_GOES_HERE
  Assert1 : xbsIndex_r4/Assert  assert_type=off  type_source=Explicitly  gui_display_data_type=Fixed-point  arith_type="Signed  (2's comp)"  n_bits=16  bin_pt=14  preci_type=Single  exp_width=8  frac_width=24  assert_rate=on  rate_source=Explicitly  period=1  output_port=on  block_type=assert
      settings: block_version=VER_STRING_GOES_HERE
  CRC Remainders1 : xbsIndex_r4/ROM  depth=256  initVector=CRC_Table32  distributed_mem=Distributed memory  rst=off  init_reg=0  en=off  latency=0  gui_display_data_type=Fixed-point  arith_type=Unsigned  n_bits=32  bin_pt=0  preci_type=Single  exp_width=8  frac_width=24  dbl_ovrd=off  optimize=Area  use_rpm=off  block_type=sprom
      settings: xl_area=[0, 0, 0, 0, 0, 0, 0]  block_version=VER_STRING_GOES_HERE
  Concat1 : xbsIndex_r4/Concat  num_inputs=2  dbl_ovrd=off  block_type=concat
      settings: block_version=VER_STRING_GOES_HERE
  Constant1 : xbsIndex_r4/Constant  const=0  gui_display_data_type=Fixed-point  arith_type=Unsigned  n_bits=8  bin_pt=0  preci_type=Single  exp_width=8  frac_width=24  explicit_period=off  period=1  equ=P=C  opselect=C  inp2=PCIN>>17  opr=+  inp1=P  carry=CIN  dbl_ovrd=off  block_type=constant
      settings: block_version=VER_STRING_GOES_HERE
  Constant3 : xbsIndex_r4/Constant  const=hex2dec('C704DD7B')  gui_display_data_type=Fixed-point  arith_type=Unsigned  n_bits=32  bin_pt=0  preci_type=Single  exp_width=8  frac_width=24  explicit_period=off  period=1  equ=P=C  opselect=C  inp2=PCIN>>17  opr=+  inp1=P  carry=CIN  dbl_ovrd=off  block_type=constant
  Endian Swap : Subsystem
  FCS Calc : Scope  NumInputPorts=8
      settings: TimeRange=10000  YMin=0~0~10~-1~-1~-1~-1~-1  YMax=1~1~120~1~1~1~1~1  SaveName=ScopeData15
  Gateway Out{1..3,10} (4 alike) : xbsIndex_r4/Gateway Out  inherit_from_input=off  hdl_port=off  timing_constraint=None  locs_specified=off  LOCs={}  UseAsDAC=off  DACChannel='1'  block_type=gatewayout
  Logical3 : xbsIndex_r4/Logical  logical_function=XOR  inputs=2  en=off  latency=0  precision=Full  arith_type=Unsigned  n_bits=8  bin_pt=2  align_bp=on  dbl_ovrd=off  block_type=logical
      settings: xl_area=[0, 0, 0, 0, 0, 0, 0]  block_version=VER_STRING_GOES_HERE
  Logical4 : xbsIndex_r4/Logical  logical_function=XOR  inputs=2  en=off  latency=0  precision=Full  arith_type=Unsigned  n_bits=8  bin_pt=2  align_bp=on  dbl_ovrd=off  block_type=logical
      settings: xl_area=[0, 0, 0, 0, 0, 0, 0]  block_version=VER_STRING_GOES_HERE
  Relational1 : xbsIndex_r4/Relational  mode=a=b  en=off  latency=0  dbl_ovrd=off  block_type=relational
  crc_accum : xbsIndex_r4/Register  init=hex2dec('FFFFFFFF')  rst=on  en=on  dbl_ovrd=off  block_type=register
      settings: xl_area=[0, 0, 0, 0, 0, 0, 0]  block_version=VER_STRING_GOES_HERE
  FCS Good : Outport
  wires:
  Endian Swap/1(I) -> Logical3/2
  Logical3/1 -> CRC Remainders1/1
  CRC Remainders1/1 -> Logical4/2
  Concat1/1 -> Logical4/1
  Constant1/1 -> Concat1/2
  Logical4/1 -> crc_accum/1
  crc_accum/1 -> Assert1/1
  Assert1/1 -> 24LSB/1, 8MSB/1, Relational1/1
  byte_valid/1 -> crc_accum/3, Gateway Out10/1
  rst/1 -> crc_accum/2, Gateway Out1/1
  24LSB/1 -> Concat1/1
  8MSB/1 -> Logical3/1
  byte/1 -> Endian Swap/1(B), Gateway Out2/1
  Constant3/1 -> Relational1/2
  Relational1/1 -> FCS Good/1, Gateway Out3/1
  Gateway Out10/1 -> FCS Calc/1  "Vin"
  Gateway Out1/1 -> FCS Calc/2  "Reset"
  Gateway Out2/1 -> FCS Calc/3  "Byte"
  Gateway Out3/1 -> FCS Calc/4  "Good"

== system wlan_phy_rx_pmd/FCS & Pkt Buf/FCS Calc/Posedge ==
  D : Inport
  Delay : xbsIndex_r4/Delay  rst=off  infoeditControl=Selection of Reset will increase slice count due to use of real FFs and instead of SRLs  en=off  latency=1  dbl_ovrd=off  reg_retiming=off  block_type=delay
      settings: infoedit=Hardware notes: A delay line is a chain, each link of which is an SRL16 followed by a flip-flop. If register retiming is enabled, the delay line is a chain of flip-flops.  xl_area=[1 1 0 0 0 0 0]  block_version=VER_STRING_GOES_HERE
  Inverter : xbsIndex_r4/Inverter  en=off  latency=0  dbl_ovrd=off  block_type=inv
      settings: xl_area=[0 0 0 0 0 0 0]  block_version=VER_STRING_GOES_HERE
  Logical1 : xbsIndex_r4/Logical  logical_function=AND  inputs=2  en=off  latency=0  precision=Full  arith_type=Unsigned  n_bits=8  bin_pt=2  align_bp=on  dbl_ovrd=off  block_type=logical
      settings: xl_area=[1 0 0 1 0 0 0]  block_version=VER_STRING_GOES_HERE
  Q : Outport
  wires:
  D/1 -> Logical1/2, Delay/1
  Logical1/1 -> Q/1
  Inverter/1 -> Logical1/1
  Delay/1 -> Inverter/1

== system wlan_phy_rx_pmd/FCS & Pkt Buf/Pkt Buf Interface/Addr Check ==
  note: The pkt buf interface logic concatenates the PHY-generated byte address
      | with the pkt buf index and MAC-specified meta-data offset. If the PHY
      | attempts to write a byte index larger than the actual pkt buf space the
      | address will wrap, overwriting the meta-data at the head of the pkt buf.
      | This subsystem prevents this by disabling the Write En for byte addresses
      | larger than a software-specified value. The MAC should set this value
      | based on its knowledge of the pkt buf size and meta-data size.
  WrEn : Inport
  Addr : Inport  Port=2
  Logical1 : xbsIndex_r4/Logical  logical_function=AND  inputs=2  en=off  latency=0  precision=Full  arith_type=Unsigned  n_bits=8  bin_pt=2  align_bp=on  dbl_ovrd=off  block_type=logical
      settings: xl_area=[0, 0, 0, 0, 0, 0, 0]  block_version=VER_STRING_GOES_HERE
  Relational : xbsIndex_r4/Relational  mode=a<b  en=off  latency=0  dbl_ovrd=off  block_type=relational
  " WrEn" : Outport
  " Addr" : Outport  Port=2
  wires:
  [regRx_PktBuf_Max_WriteAddr] -> Relational/2
  Relational/1 -> Logical1/2
  WrEn/1 -> Logical1/1
  Logical1/1 -> " WrEn"/1
  Addr/1 -> Relational/1, " Addr"/1
